simulink model "TosAeb"  (R2021a)
settings: Solver=FixedStepDiscrete  StartTime=0.0  StopTime=inf  FixedStep=0.01  RelTol=1e-3
undefined symbols (not defined in the file or in its folder): ACCModSts, AccSts1, AEB_ObjectMovingOrientation, AEB_ObjectMovingState, CN_BTCActive, CN_CurrentGearR, CN_CurrentGearR_DHT, CN_PTCActive, CN_VDCActive, CN_VSC_EspOff, CN_VSC_FCW_AEB_Error, CN_VSC_FCW_AEB_NoDispay, CN_VSC_FCW_AEB_PerfmDgdtn, CN_VSC_FCW_AEB_TempUnAvlbl, CN_VSC_VehStandstill, CNaccelAvoidMax, CNaccelAvoidMin, CNbrakePedalPress, CNcomfortStrLeftAccelMax, CNcomfortStrLeftAccelMin, CNcomfortStrRightAccelMax, CNcomfortStrRightAccelMin, CNDrvDoorOpen, CNDrvModeEco, CNDrvModeNorm, CNDrvModeOther, CNDrvModeSport, CNDrvSBRreminder, CNNull, CNobjInvalidID, CNPassDoorOpen, CNPassSBRreminder, CNPEBSAccelNegInf, CNPEBSAccelNull, CNPEBSTimeInf, CNPEBSTimeNull, CNSTOPMAX, CNSTOPNULL, CNsubjWidth, CNSwtOFF, CNSwtON, CNTTCMAX, CNTTCNULL, CNVehConfigAEBCycNone, CNVehConfigAEBNone, CNVehConfigAEBPedNone, CNVehConfigFCWNone, CNVehConfigJANone, CollisionSeverity, FALSE, FCWSnvty, JACycObjCalcInfoDefaultParams, JAPedObjCalcInfoDefaultParams, JAVehObjCalcInfoDefaultParams, latCycObjCalcInfoDefaultParams, latPedObjCalcInfoDefaultParams, longObjCalcInfoDefaultParams, ModSts, movingStatus, ObjectType ... (+1112)
libraries: simulink x3031, AEBSFunctionLib x50, Ta_Library x33, AEBSStateMachineLib x23, Mylibrary x12, simulink_extras x7, ObjSelect_lib x3, dspstat3 x2
(part 42 of 102)

== system TosAeb/FcwAebJa_Step_sys/ALG_AEBSystem/ALG_AEBController/AEB_FuncCondCalcApp/LongFunctionCondCalc/vehLatentWarningSuppAborCondFulfilled/vehLatentWarningAborCondCalc/vehLatentWarningSituationRelAborCondCalc ==
  subjVel : Inport
  dxFrontBumper : Inport  Port=2
  Compare To Constant : simulink/Logic and Bit Operations/Compare To Constant  relop===  const=0  OutDataTypeStr=boolean
  Compare To Constant1 : simulink/Logic and Bit Operations/Compare To Constant  relop=>  const=P_AEB_Func_vehLatentWarningTimeGapCounterThres  OutDataTypeStr=boolean
  Compare To Constant2 : simulink/Logic and Bit Operations/Compare To Constant  relop=>  const=P_AEB_Func_vehLatentWarningTimeGapDurationAborThres  OutDataTypeStr=boolean
  If : If  IfExpression=u1
  If Action Subsystem : Subsystem  TreatAsAtomicUnit=on
  If Action Subsystem1 : Subsystem  TreatAsAtomicUnit=on
  Logical Operator2 : Logic  AllPortsSameDT=off  OutDataTypeStr=boolean
  Merge : Merge
  timer : Subsystem
  vehLatentWarningSituationRelAborCondFulfilled : Outport
  wires:
  Logical Operator2/1 -> vehLatentWarningSituationRelAborCondFulfilled/1
  Compare To Constant1/1 -> timer/1(cond), Logical Operator2/1
  If/2 -> If Action Subsystem1/Action
  If Action Subsystem/1(latentWarningTimeGap) -> Merge/1
  If Action Subsystem1/1(latentWarningTimeGap) -> Merge/2
  dxFrontBumper/1 -> If Action Subsystem1/1(dxFrontBumper)
  subjVel/1 -> If Action Subsystem1/2(subjVel), Compare To Constant/1
  Compare To Constant/1 -> If/1
  If/1 -> If Action Subsystem/Action
  Merge/1 -> Compare To Constant1/1
  timer/1(time) -> Compare To Constant2/1
  Compare To Constant2/1 -> Logical Operator2/2

== system TosAeb/FcwAebJa_Step_sys/ALG_AEBSystem/ALG_AEBController/AEB_FuncCondCalcApp/LongFunctionCondCalc/vehLatentWarningSuppAborCondFulfilled/vehLatentWarningAborCondCalc/vehLatentWarningVehRelActiveCondCalc ==
  vehStateCalcLatentWarningAborFlg : Inport
  subjVel : Inport  Port=2
  ACCModSts : Inport  Port=3
  ACCStateJudge : Subsystem
  vehLatentWarningVehRelAborCondJudge : Subsystem
  vehLatentWarningVehRelAborCondFulfilled : Outport
  wires:
  ACCModSts/1 -> ACCStateJudge/1(ACCModSts)
  subjVel/1 -> vehLatentWarningVehRelAborCondJudge/2(subjVel)
  vehLatentWarningVehRelAborCondJudge/1(vehLatentWarningVehRelAborCondFulfilled) -> vehLatentWarningVehRelAborCondFulfilled/1
  vehStateCalcLatentWarningAborFlg/1 -> vehLatentWarningVehRelAborCondJudge/1(vehStateCalcLatentWarningAborFlg)
  ACCStateJudge/1(ACCStateSuppCondFlg) -> vehLatentWarningVehRelAborCondJudge/3(ACCStateSuppCondFlg)

== system TosAeb/FcwAebJa_Step_sys/ALG_AEBSystem/ALG_AEBController/AEB_FuncCondCalcApp/LongFunctionCondCalc/vehLatentWarningSuppAborCondFulfilled/vehLatentWarningSuppCondCalc/vehLatentWarningObjRelActiveCondCalc ==
  ObjectMovingState : Inport
  ObjectType : Inport  Port=2
  ObjectMovingOrientation : Inport  Port=3
  ObjectID : Inport  Port=4
  ObjectExistProb : Inport  Port=5
  ObjectIsObstacleProb : Inport  Port=6
  Compare To Constant : simulink/Logic and Bit Operations/Compare To Constant  relop===  const=AEB_ObjectMovingState.Still  OutDataTypeStr=boolean
  Compare To Constant1 : simulink/Logic and Bit Operations/Compare To Constant  relop===  const=AEB_ObjectMovingState.Moving  OutDataTypeStr=boolean
  Compare To Constant12 : simulink/Logic and Bit Operations/Compare To Constant  relop===  const=FALSE  OutDataTypeStr=boolean
  Compare To Constant14 : simulink/Logic and Bit Operations/Compare To Constant  relop===  const=ObjectTypeCopy.PEDESTRIAN  OutDataTypeStr=boolean
  Compare To Constant15 : simulink/Logic and Bit Operations/Compare To Constant  relop===  const=ObjectTypeCopy.BICYCLE  OutDataTypeStr=boolean
  Compare To Constant2 : simulink/Logic and Bit Operations/Compare To Constant  relop===  const=AEB_ObjectMovingState.Stopped  OutDataTypeStr=boolean
  Compare To Constant3 : simulink/Logic and Bit Operations/Compare To Constant  relop===  const=AEB_ObjectMovingOrientation.SameDir  OutDataTypeStr=boolean
  Compare To Constant4 : simulink/Logic and Bit Operations/Compare To Constant  relop=~=  const=CNobjInvalidID  OutDataTypeStr=boolean
  Compare To Constant5 : simulink/Logic and Bit Operations/Compare To Constant  relop=>  const=P_AEB_Func_vehLatentWarningObjObstacleProbActivationThres  OutDataTypeStr=boolean
  Compare To Constant6 : simulink/Logic and Bit Operations/Compare To Constant  relop=~=  const=CNobjInvalidID  OutDataTypeStr=boolean
  Logical Operator : Logic  Operator=OR  Inputs=3  AllPortsSameDT=off  OutDataTypeStr=boolean
  Logical Operator1 : Logic  AllPortsSameDT=off  OutDataTypeStr=boolean
  Logical Operator2 : Logic  AllPortsSameDT=off  OutDataTypeStr=boolean
  Logical Operator5 : Logic  Inputs=5  AllPortsSameDT=off  OutDataTypeStr=boolean
  Logical Operator6 : Logic  Operator=OR  AllPortsSameDT=off  OutDataTypeStr=boolean
  Product : Product
  Relational Operator : RelationalOperator  Operator===  OutDataTypeStr=boolean  RndMeth=Simplest
  Unit Delay : UnitDelay  SampleTime=-1  HasFrameUpgradeWarning=on
  vehLatentWarningObjRelActiveCondFulfilled : Outport
  wires:
  Compare To Constant15/1 -> Logical Operator6/2
  Compare To Constant14/1 -> Logical Operator6/1
  ObjectMovingState/1 -> Compare To Constant/1, Compare To Constant2/1, Compare To Constant1/1
  Compare To Constant2/1 -> Logical Operator/2
  Compare To Constant1/1 -> Logical Operator/1
  ObjectMovingOrientation/1 -> Compare To Constant3/1
  ObjectID/1 -> Compare To Constant6/1, Unit Delay/1, Relational Operator/1, Compare To Constant4/1
  Unit Delay/1 -> Relational Operator/2
  ObjectExistProb/1 -> Product/1
  ObjectIsObstacleProb/1 -> Product/2
  Compare To Constant6/1 -> Logical Operator2/1
  Compare To Constant5/1 -> Logical Operator2/2
  Product/1 -> Compare To Constant5/1
  Compare To Constant/1 -> Logical Operator/3
  Relational Operator/1 -> Logical Operator1/2
  Compare To Constant4/1 -> Logical Operator1/1
  Logical Operator5/1 -> vehLatentWarningObjRelActiveCondFulfilled/1  "vehPreWarningObjRelActiveCondFulfilled"
  Compare To Constant3/1 -> Logical Operator5/3  "objMovingOrientationCondFulfilled"
  Logical Operator2/1 -> Logical Operator5/5  "objQualityCondFulfilled"
  Logical Operator6/1 -> Compare To Constant12/1  "pedCycObjCondSuppFlg"
  Logical Operator/1 -> Logical Operator5/1  "objMovingStateCondFulfilled"
  Logical Operator1/1 -> Logical Operator5/4  "objIDCondFulfilled"
  ObjectType/1 -> Compare To Constant14/1, Compare To Constant15/1
  Compare To Constant12/1 -> Logical Operator5/2  "pedCycReleventCondFulfilled"

== system TosAeb/FcwAebJa_Step_sys/ALG_AEBSystem/ALG_AEBController/AEB_FuncCondCalcApp/LongFunctionCondCalc/vehLatentWarningSuppAborCondFulfilled/vehLatentWarningSuppCondCalc/vehLatentWarningSituationRelActiveCondCalc ==
  subjVel : Inport
  dxFrontBumper : Inport  Port=2
  FCWSnvty : Inport  Port=3
  Compare To Constant : simulink/Logic and Bit Operations/Compare To Constant  relop=<  const=0.1  OutDataTypeStr=boolean
  Constant : Constant  Value=Ts
  Constant1 : Constant  Value=30
  If : If  IfExpression=u1
  If Action Subsystem : Subsystem  TreatAsAtomicUnit=on
  If Action Subsystem1 : Subsystem  TreatAsAtomicUnit=on
  Logical Operator2 : Logic  AllPortsSameDT=off  OutDataTypeStr=boolean
  Merge : Merge
  Relational Operator : RelationalOperator  Operator=<  OutDataTypeStr=boolean  RndMeth=Simplest
  Relational Operator1 : RelationalOperator  Operator=>  OutDataTypeStr=boolean  RndMeth=Simplest
  TimeThesCalc : Subsystem
  timer1 : AEBSFunctionLib/timer  SystemSampleTime=-1  VariantActivationTime=update diagram  Latency=0  AutoFrameSizeCalculation=off
  vehLatentWarningSituationRelActiveCondFulfilled : Outport
  wires:
  Constant1/1 -> timer1/3
  Constant/1 -> timer1/2
  TimeThesCalc/2(prolongTime) -> Relational Operator1/2
  timer1/1 -> Relational Operator1/1
  TimeThesCalc/1(timeGapThes) -> Relational Operator/2
  Merge/1 -> Relational Operator/1
  FCWSnvty/1 -> TimeThesCalc/1(FCWSnvty)
  Relational Operator1/1 -> Logical Operator2/2
  Relational Operator/1 -> Logical Operator2/1, timer1/1
  If Action Subsystem1/1(latentWarningTimeGap) -> Merge/2
  If Action Subsystem/1(latentWarningTimeGap) -> Merge/1
  subjVel/1 -> If Action Subsystem1/2(subjVel), Compare To Constant/1
  dxFrontBumper/1 -> If Action Subsystem1/1(dxFrontBumper)
  If/2 -> If Action Subsystem1/Action
  If/1 -> If Action Subsystem/Action
  Compare To Constant/1 -> If/1
  Logical Operator2/1 -> vehLatentWarningSituationRelActiveCondFulfilled/1  "vehLatentWarningSituationRelActiveCondFulfilled"

== system TosAeb/FcwAebJa_Step_sys/ALG_AEBSystem/ALG_AEBController/AEB_FuncCondCalcApp/LongFunctionCondCalc/vehLatentWarningSuppAborCondFulfilled/vehLatentWarningSuppCondCalc/vehLatentWarningSuppCondFinalJudge ==
  vehLatentWarningObjRelActiveCondFulfilled : Inport
  vehLatentWarningVehRelActiveCondFulfilled : Inport  Port=2
  vehLatentWarningSituationRelActiveCondFulfilled : Inport  Port=3
  Compare To Constant : simulink/Logic and Bit Operations/Compare To Constant  relop===  const=FALSE  OutDataTypeStr=boolean
  Compare To Constant1 : simulink/Logic and Bit Operations/Compare To Constant  relop===  const=FALSE  OutDataTypeStr=boolean
  Compare To Constant2 : simulink/Logic and Bit Operations/Compare To Constant  relop===  const=FALSE  OutDataTypeStr=boolean
  Logical Operator : Logic  Operator=OR  Inputs=3  AllPortsSameDT=off  OutDataTypeStr=boolean
  vehLatentWarningAborCondFulfilled : Outport
  wires:
  Compare To Constant2/1 -> Logical Operator/3
  Compare To Constant1/1 -> Logical Operator/2
  Compare To Constant/1 -> Logical Operator/1
  vehLatentWarningVehRelActiveCondFulfilled/1 -> Compare To Constant1/1
  vehLatentWarningObjRelActiveCondFulfilled/1 -> Compare To Constant/1
  vehLatentWarningSituationRelActiveCondFulfilled/1 -> Compare To Constant2/1
  Logical Operator/1 -> vehLatentWarningAborCondFulfilled/1

== system TosAeb/FcwAebJa_Step_sys/ALG_AEBSystem/ALG_AEBController/AEB_FuncCondCalcApp/LongFunctionCondCalc/vehLatentWarningSuppAborCondFulfilled/vehLatentWarningSuppCondCalc/vehLatentWarningVehRelActiveCondCalc ==
  subjVel : Inport
  ACCModSts : Inport  Port=2
  vehStateCalcLatentWarningSuppFlg : Inport  Port=3
  ACCStateJudge : Subsystem
  subjVelWork : Subsystem
  vehLatentWarningVehRelActiveCondJudge : Subsystem
  vehLatentWarningVehRelActiveCondFulfilled : Outport
  wires:
  ACCModSts/1 -> ACCStateJudge/1(ACCModSts)
  subjVelWork/1(subjVelWorkMax) -> vehLatentWarningVehRelActiveCondJudge/2(subjVelWorkMax)
  subjVelWork/2(subjVelWorkMin) -> vehLatentWarningVehRelActiveCondJudge/3(subjVelWorkMin)
  ACCStateJudge/1(ACCStateSuppCondFlg) -> vehLatentWarningVehRelActiveCondJudge/4(ACCStateSuppCondFlg)
  subjVel/1 -> vehLatentWarningVehRelActiveCondJudge/1(subjVel)
  vehStateCalcLatentWarningSuppFlg/1 -> vehLatentWarningVehRelActiveCondJudge/5(vehStateCalcLatentWarningSuppFlg)
  vehLatentWarningVehRelActiveCondJudge/1(vehLatentWarningVehRelActiveCondFulfilled) -> vehLatentWarningVehRelActiveCondFulfilled/1

== system TosAeb/FcwAebJa_Step_sys/ALG_AEBSystem/ALG_AEBController/AEB_FuncCondCalcApp/LongFunctionCondCalc/vehPreWarningSuppAborCondFulfilled/vehPreWarningAborCondCalc/vehPreWarningAborCondFinalJudge ==
  vehPreWarningObjRelAborCondFulfilled : Inport
  vehPreWarningVehRelAborCondFulfilled : Inport  Port=2
  vehPreWarningSituationRelAborCondFulfilled : Inport  Port=3
  Compare To Constant : simulink/Logic and Bit Operations/Compare To Constant  relop===  const=TRUE  OutDataTypeStr=boolean
  Compare To Constant1 : simulink/Logic and Bit Operations/Compare To Constant  relop===  const=TRUE  OutDataTypeStr=boolean
  Compare To Constant2 : simulink/Logic and Bit Operations/Compare To Constant  relop===  const=TRUE  OutDataTypeStr=boolean
  Logical Operator : Logic  Operator=OR  Inputs=3  AllPortsSameDT=off  OutDataTypeStr=boolean
  vehPreWarningAborCond : Outport
  wires:
  Compare To Constant2/1 -> Logical Operator/3
  Compare To Constant1/1 -> Logical Operator/2
  Compare To Constant/1 -> Logical Operator/1
  Logical Operator/1 -> vehPreWarningAborCond/1
  vehPreWarningSituationRelAborCondFulfilled/1 -> Compare To Constant2/1
  vehPreWarningObjRelAborCondFulfilled/1 -> Compare To Constant/1
  vehPreWarningVehRelAborCondFulfilled/1 -> Compare To Constant1/1

== system TosAeb/FcwAebJa_Step_sys/ALG_AEBSystem/ALG_AEBController/AEB_FuncCondCalcApp/LongFunctionCondCalc/vehPreWarningSuppAborCondFulfilled/vehPreWarningAborCondCalc/vehPreWarningObjRelAborCondCalc ==
  ObjectID : Inport
  Compare To Constant4 : simulink/Logic and Bit Operations/Compare To Constant  relop===  const=CNobjInvalidID  OutDataTypeStr=boolean
  Logical Operator1 : Logic  Operator=OR  AllPortsSameDT=off  OutDataTypeStr=boolean
  Relational Operator : RelationalOperator  Operator=~=  OutDataTypeStr=boolean  RndMeth=Simplest
  Unit Delay : UnitDelay  SampleTime=-1  HasFrameUpgradeWarning=on
  vehPreWarningObjRelAborCondFulfilled : Outport
  wires:
  ObjectID/1 -> Unit Delay/1, Relational Operator/1, Compare To Constant4/1
  Unit Delay/1 -> Relational Operator/2
  Relational Operator/1 -> Logical Operator1/2
  Compare To Constant4/1 -> Logical Operator1/1
  Logical Operator1/1 -> vehPreWarningObjRelAborCondFulfilled/1  "vehPreWarningObjRelAborCondFulfilled"

== system TosAeb/FcwAebJa_Step_sys/ALG_AEBSystem/ALG_AEBController/AEB_FuncCondCalcApp/LongFunctionCondCalc/vehPreWarningSuppAborCondFulfilled/vehPreWarningAborCondCalc/vehPreWarningSituationRelAborCondCalc ==
  longCollisionSeverity : Inport
  Compare To Constant : simulink/Logic and Bit Operations/Compare To Constant  relop=~=  const=CollisionSeverity.Low  OutDataTypeStr=boolean
  Compare To Constant1 : simulink/Logic and Bit Operations/Compare To Constant  relop=~=  const=CollisionSeverity.Medium  OutDataTypeStr=boolean
  Compare To Constant2 : simulink/Logic and Bit Operations/Compare To Constant  relop=~=  const=CollisionSeverity.High  OutDataTypeStr=boolean
  Logical Operator : Logic  Inputs=3  AllPortsSameDT=off  OutDataTypeStr=boolean
  vehPreWarningSituationRelAborCondFulfilled : Outport
  wires:
  longCollisionSeverity/1 -> Compare To Constant/1, Compare To Constant2/1, Compare To Constant1/1
  Compare To Constant/1 -> Logical Operator/1
  Compare To Constant1/1 -> Logical Operator/2
  Compare To Constant2/1 -> Logical Operator/3
  Logical Operator/1 -> vehPreWarningSituationRelAborCondFulfilled/1  "vehPreWarningSituationRelAborCondFulfilled"

== system TosAeb/FcwAebJa_Step_sys/ALG_AEBSystem/ALG_AEBController/AEB_FuncCondCalcApp/LongFunctionCondCalc/vehPreWarningSuppAborCondFulfilled/vehPreWarningAborCondCalc/vehPreWarningVehRelActiveCondCalc ==
  SteerWheelAngle : Inport
  SteerWheelSpd : Inport  Port=2
  ESPmasterCylindBrakePress : Inport  Port=3
  vehStateCalcPreWarningAborFlg : Inport  Port=4
  EBAFuncImpArb : Inport  Port=5
  DriverCondJudge : Subsystem
  vehPreWarningVehRelAborCondJudge : Subsystem
  vehPreWarningVehRelAborCondFulfilled : Outport
  wires:
  EBAFuncImpArb/1 -> vehPreWarningVehRelAborCondJudge/3(EBAFuncImpArb)
  SteerWheelAngle/1 -> DriverCondJudge/1(SteerWheelAngle)
  SteerWheelSpd/1 -> DriverCondJudge/2(SteerWheelSpd)
  ESPmasterCylindBrakePress/1 -> DriverCondJudge/3(AccrPedlPosnDiagc)
  DriverCondJudge/1(driverAborCondFlg) -> vehPreWarningVehRelAborCondJudge/1(driverAborCondFlg)
  vehStateCalcPreWarningAborFlg/1 -> vehPreWarningVehRelAborCondJudge/2(vehStateCalcPreWarningAborFlg)
  vehPreWarningVehRelAborCondJudge/1(vehPreWarningVehRelAborCondFulfilled) -> vehPreWarningVehRelAborCondFulfilled/1  "vehPreWarningVehRelAborCondFulfilled"

== system TosAeb/FcwAebJa_Step_sys/ALG_AEBSystem/ALG_AEBController/AEB_FuncCondCalcApp/LongFunctionCondCalc/vehPreWarningSuppAborCondFulfilled/vehPreWarningSuppCondCalc/vehPreWarningObjRelActiveCondCalc ==
  ObjectMovingState : Inport
  dz : Inport  Port=2
  ObjectTypeCameraConfirmed : Inport  Port=3
  AEB_PedResp : Inport  Port=4
  ObjectType : Inport  Port=5  OutDataTypeStr=Enum: ObjectTypeCopy
  subjCurvTraj : Inport  Port=6
  subjLatAccel : Inport  Port=7
  subjVel : Inport  Port=8
  vehConfigAEBCyc : Inport  Port=9
  vehConfigAEBPed : Inport  Port=10
  ObjectMovingOrientation : Inport  Port=11
  ObjectID : Inport  Port=12
  ObjectExistProb : Inport  Port=13
  ObjectIsObstacleProb : Inport  Port=14
  dxVariance : Inport  Port=15
  dxFrontBumper : Inport  Port=16
  ObjFusQuality : Inport  Port=17
  Compare To Constant : simulink/Logic and Bit Operations/Compare To Constant  relop===  const=AEB_ObjectMovingState.Still  OutDataTypeStr=boolean
  Compare To Constant1 : simulink/Logic and Bit Operations/Compare To Constant  relop===  const=AEB_ObjectMovingState.Moving  OutDataTypeStr=boolean
  Compare To Constant10 : simulink/Logic and Bit Operations/Compare To Constant  relop=<  const=P_AEB_Func_vehPreWarningDxActivationThres  OutDataTypeStr=boolean
  Compare To Constant11 : simulink/Logic and Bit Operations/Compare To Constant  relop===  const=TRUE  OutDataTypeStr=boolean
  Compare To Constant12 : simulink/Logic and Bit Operations/Compare To Constant  relop===  const=FALSE  OutDataTypeStr=boolean
  Compare To Constant14 : simulink/Logic and Bit Operations/Compare To Constant  relop===  const=AEB_ObjectMovingState.Still  OutDataTypeStr=boolean
  Compare To Constant2 : simulink/Logic and Bit Operations/Compare To Constant  relop===  const=AEB_ObjectMovingState.Stopped  OutDataTypeStr=boolean
  Compare To Constant3 : simulink/Logic and Bit Operations/Compare To Constant  relop===  const=AEB_ObjectMovingOrientation.SameDir  OutDataTypeStr=boolean
  Compare To Constant4 : simulink/Logic and Bit Operations/Compare To Constant  relop=~=  const=CNobjInvalidID  OutDataTypeStr=boolean
  Compare To Constant5 : simulink/Logic and Bit Operations/Compare To Constant  relop=>  const=P_AEB_Func_vehPreWarningObjObstacleProbActivationThres  OutDataTypeStr=boolean
  Compare To Constant6 : simulink/Logic and Bit Operations/Compare To Constant  relop=~=  const=CNobjInvalidID  OutDataTypeStr=boolean
  Compare To Constant7 : simulink/Logic and Bit Operations/Compare To Constant  relop===  const=TRUE  OutDataTypeStr=boolean
  Compare To Constant8 : simulink/Logic and Bit Operations/Compare To Constant  relop=<  const=P_AEB_Func_vehPreWarningObjHeightActivationThres  OutDataTypeStr=boolean
  Compare To Constant9 : simulink/Logic and Bit Operations/Compare To Constant  relop=<  const=P_AEB_Func_vehPreWarningDxVarActivationThres  OutDataTypeStr=boolean
  Constant : Constant  Value=TRUE
  Constant1 : Constant  Value=P_AEB_Func_vehPreWarningSubjLatAccelActivationThresForPedCyc
  Constant2 : Constant  Value=P_AEB_Func_vehPreWarningSubjCurvTrajActivationThresForPedCyc
  Constant3 : Constant  Value=P_AEB_Func_vehPreWarningSubjVelActivationThresForPedCyc
  Logical Operator : Logic  Operator=OR  Inputs=3  AllPortsSameDT=off  OutDataTypeStr=boolean
  Logical Operator1 : Logic  AllPortsSameDT=off  OutDataTypeStr=boolean
  Logical Operator2 : Logic  AllPortsSameDT=off  OutDataTypeStr=boolean
  Logical Operator5 : Logic  Inputs=10  AllPortsSameDT=off  OutDataTypeStr=boolean
  PedCycObjCondSuppJudgeFlow : AEBSFunctionLib/PedCycObjCondSuppJudgeFlow  SystemSampleTime=-1  VariantActivationTime=update diagram  Latency=0  AutoFrameSizeCalculation=off
  Product : Product
  Relational Operator : RelationalOperator  Operator===  OutDataTypeStr=boolean  RndMeth=Simplest
  Switch : Switch  Criteria=u2 ~= 0
  Unit Delay : UnitDelay  SampleTime=-1  HasFrameUpgradeWarning=on
  vehPreWarningObjRelActiveCondFulfilled : Outport
  wires:
  Compare To Constant11/1 -> Logical Operator5/10  "ObjFusQuality"
  ObjFusQuality/1 -> Compare To Constant11/1
  subjLatAccel/1 -> PedCycObjCondSuppJudgeFlow/4
  subjVel/1 -> PedCycObjCondSuppJudgeFlow/5
  vehConfigAEBCyc/1 -> PedCycObjCondSuppJudgeFlow/6
  vehConfigAEBPed/1 -> PedCycObjCondSuppJudgeFlow/7
  AEB_PedResp/1 -> PedCycObjCondSuppJudgeFlow/8
  Constant3/1 -> PedCycObjCondSuppJudgeFlow/9
  Constant2/1 -> PedCycObjCondSuppJudgeFlow/10
  Constant1/1 -> PedCycObjCondSuppJudgeFlow/11
  subjCurvTraj/1 -> PedCycObjCondSuppJudgeFlow/3
  ObjectType/1 -> PedCycObjCondSuppJudgeFlow/2
  Constant/1 -> Switch/3
  Compare To Constant14/1 -> Switch/2
  Compare To Constant8/1 -> Switch/1
  Compare To Constant7/1 -> Logical Operator5/3  "FusionCondFulfilled"
  Compare To Constant12/1 -> Logical Operator5/4  "pedCycReleventCondFulfilled"
  ObjectTypeCameraConfirmed/1 -> Compare To Constant7/1, PedCycObjCondSuppJudgeFlow/1
  Compare To Constant9/1 -> Logical Operator5/8  "dxVarCondFulfilled"
  Logical Operator1/1 -> Logical Operator5/6  "objIDCondFulfilled"
  Logical Operator/1 -> Logical Operator5/1  "objMovingStateCondFulfilled"
  Compare To Constant10/1 -> Logical Operator5/9  "dxRelCondFulfilled"
  PedCycObjCondSuppJudgeFlow/1 -> Compare To Constant12/1  "pedCycObjCondSuppFlg"
  Logical Operator2/1 -> Logical Operator5/7  "objQualityCondFulfilled"
  Compare To Constant3/1 -> Logical Operator5/5  "objMovingOrientationCondFulfilled"
  Switch/1 -> Logical Operator5/2  "objHeightInfoFulfilled"
  Logical Operator5/1 -> vehPreWarningObjRelActiveCondFulfilled/1  "vehPreWarningObjRelActiveCondFulfilled"
  Compare To Constant4/1 -> Logical Operator1/1
  Relational Operator/1 -> Logical Operator1/2
  Compare To Constant/1 -> Logical Operator/3
  dz/1 -> Compare To Constant8/1
  dxVariance/1 -> Compare To Constant9/1
  dxFrontBumper/1 -> Compare To Constant10/1
  Product/1 -> Compare To Constant5/1
  Compare To Constant5/1 -> Logical Operator2/2
  Compare To Constant6/1 -> Logical Operator2/1
  ObjectIsObstacleProb/1 -> Product/2
  ObjectExistProb/1 -> Product/1
  Unit Delay/1 -> Relational Operator/2
  ObjectID/1 -> Compare To Constant4/1, Relational Operator/1, Unit Delay/1, Compare To Constant6/1
  ObjectMovingOrientation/1 -> Compare To Constant3/1
  Compare To Constant1/1 -> Logical Operator/1
  Compare To Constant2/1 -> Logical Operator/2
  ObjectMovingState/1 -> Compare To Constant14/1, Compare To Constant/1, Compare To Constant2/1, Compare To Constant1/1

== system TosAeb/FcwAebJa_Step_sys/ALG_AEBSystem/ALG_AEBController/AEB_FuncCondCalcApp/LongFunctionCondCalc/vehPreWarningSuppAborCondFulfilled/vehPreWarningSuppCondCalc/vehPreWarningSituationRelActiveCondCalc ==
  longCollisionSeverity : Inport  OutDataTypeStr=Enum: CollisionSeverity
  overlapRatio : Inport  Port=2
  dxFrontBumper : Inport  Port=3
  probEscape : Inport  Port=4
  subjCurvTraj : Inport  Port=5
  TTC : Inport  Port=6
  FcwHMISetting : Inport  Port=7
  subjVel : Inport  Port=8  OutDataTypeStr=single
  ACCModSts : Inport  Port=9
  AEB_Func_VehPreWarning_TTC_Thres1 : Lookup_n-D  Commented=on  NumberOfTableDimensions=1  BreakpointsForDimension1=P_AEB_Func_vehPreWarning_TTC_ThresX  Table=P_AEB_Func_vehPreWarning_TTC_ThresY  ExtrapMethod=Clip  InternalRulePriority=Speed  RndMeth=Simplest  InputSameDT=on
  IsTtcCondFulfilledCalc : Subsystem
  Subsystem1 : Subsystem
  vehPreWarningSituationRelActiveCondFulfilled : Outport
  wires:
  TTC/1 -> IsTtcCondFulfilledCalc/1(TTC)
  subjVel/1 -> IsTtcCondFulfilledCalc/3(subjVel)
  FcwHMISetting/1 -> IsTtcCondFulfilledCalc/2(FcwHMISetting)
  longCollisionSeverity/1 -> Subsystem1/1(longCollisionSeverity)
  overlapRatio/1 -> Subsystem1/2(overlapRatio)
  dxFrontBumper/1 -> Subsystem1/3(dxFrontBumper)
  probEscape/1 -> Subsystem1/4(probEscape)
  subjCurvTraj/1 -> Subsystem1/5(subjCurvTraj)
  IsTtcCondFulfilledCalc/1(IsTtcCondFulfilled) -> Subsystem1/6(IsTtcCondFulfilled)  "IsTtcCondFulfilled"
  Subsystem1/1(vehPreWarningSituationRelActiveCondFulfilled) -> vehPreWarningSituationRelActiveCondFulfilled/1
  ACCModSts/1 -> IsTtcCondFulfilledCalc/4(ACCModSts)

== system TosAeb/FcwAebJa_Step_sys/ALG_AEBSystem/ALG_AEBController/AEB_FuncCondCalcApp/LongFunctionCondCalc/vehPreWarningSuppAborCondFulfilled/vehPreWarningSuppCondCalc/vehPreWarningSuppCondFinalJudge ==
  vehPreWarningObjRelActiveCondFulfilled : Inport
  vehPreWarningVehRelActiveCondFulfilled : Inport  Port=2
  vehPreWarningSituationRelActiveCondFulfilled : Inport  Port=3
  Compare To Constant1 : simulink/Logic and Bit Operations/Compare To Constant  relop===  const=FALSE  OutDataTypeStr=boolean
  Compare To Constant2 : simulink/Logic and Bit Operations/Compare To Constant  relop===  const=FALSE  OutDataTypeStr=boolean
  Compare To Constant5 : simulink/Logic and Bit Operations/Compare To Constant  relop===  const=FALSE  OutDataTypeStr=boolean
  Logical Operator : Logic  Operator=OR  Inputs=3  AllPortsSameDT=off  OutDataTypeStr=boolean
  vehPreWarningSuppCond : Outport
  wires:
  Compare To Constant2/1 -> Logical Operator/3
  Compare To Constant1/1 -> Logical Operator/2
  Compare To Constant5/1 -> Logical Operator/1
  Logical Operator/1 -> vehPreWarningSuppCond/1
  vehPreWarningSituationRelActiveCondFulfilled/1 -> Compare To Constant2/1
  vehPreWarningObjRelActiveCondFulfilled/1 -> Compare To Constant5/1
  vehPreWarningVehRelActiveCondFulfilled/1 -> Compare To Constant1/1

== system TosAeb/FcwAebJa_Step_sys/ALG_AEBSystem/ALG_AEBController/AEB_FuncCondCalcApp/LongFunctionCondCalc/vehPreWarningSuppAborCondFulfilled/vehPreWarningSuppCondCalc/vehPreWarningVehRelActiveCondCalc ==
  subjVel : Inport
  ObjectMovingState : Inport  Port=2
  vehStateCalcPreWarningSuppFlg : Inport  Port=3
  SteerWheelAngle : Inport  Port=4
  SteerWheelSpd : Inport  Port=5
  VehConfigIbooster : Inport  Port=6
  ESPmasterCylindBrakePress : Inport  Port=7
  BrakePedalStatus_G : Inport  Port=8
  IB_BrkPedalAppld : Inport  Port=9
  AccrPedlPosnDiagc : Inport  Port=10
  DriverCondJudge : Subsystem
  subjVelWork : Subsystem
  vehPreWarningVehRelActiveCondJudge : Subsystem
  vehPreWarningVehRelActiveCondFulfilled : Outport
  wires:
  VehConfigIbooster/1 -> DriverCondJudge/3(VehConfigIbooster)
  IB_BrkPedalAppld/1 -> DriverCondJudge/6(IB_BrkPedalAppld)
  AccrPedlPosnDiagc/1 -> DriverCondJudge/7(AccrPedlPosnDiagc)
  BrakePedalStatus_G/1 -> DriverCondJudge/5(BrakePedalStatus_G)
  ESPmasterCylindBrakePress/1 -> DriverCondJudge/4(ESPmasterCylindBrakePress)
  SteerWheelSpd/1 -> DriverCondJudge/2(SteerWheelSpd)
  SteerWheelAngle/1 -> DriverCondJudge/1(SteerWheelAngle)
  vehPreWarningVehRelActiveCondJudge/1(vehPreWarningVehRelActiveCondFulfilled) -> vehPreWarningVehRelActiveCondFulfilled/1  "vehPreWarningVehRelActiveCondFulfilled"
  vehStateCalcPreWarningSuppFlg/1 -> vehPreWarningVehRelActiveCondJudge/5(vehStateCalcPreWarningSuppFlg)
  subjVel/1 -> vehPreWarningVehRelActiveCondJudge/1(subjVel)
  DriverCondJudge/1(driverSuppCondFlg) -> vehPreWarningVehRelActiveCondJudge/4(driverSuppCondFlg)
  subjVelWork/2(subjVelWorkMin) -> vehPreWarningVehRelActiveCondJudge/3(subjVelWorkMin)
  subjVelWork/1(subjVelWorkMax) -> vehPreWarningVehRelActiveCondJudge/2(subjVelWorkMax)
  ObjectMovingState/1 -> subjVelWork/1(ObjectMovingState)

== system TosAeb/FcwAebJa_Step_sys/ALG_AEBSystem/ALG_AEBController/AEB_FuncCondCalcApp/LongFunctionCondCalc/vehPrefillSuppAborCondCalc/vehPrefillAborCondCalc/vehPrefillAborCondFinalJudge ==
  vehPrefillObjRelAborCondFulfilled : Inport
  vehPrefillVehRelAborCondFulfilled : Inport  Port=2
  vehPrefillSituationRelAborCondFulfilled : Inport  Port=3
  Compare To Constant1 : simulink/Logic and Bit Operations/Compare To Constant  relop===  const=TRUE  OutDataTypeStr=boolean
  Compare To Constant2 : simulink/Logic and Bit Operations/Compare To Constant  relop===  const=TRUE  OutDataTypeStr=boolean
  Compare To Constant3 : simulink/Logic and Bit Operations/Compare To Constant  relop===  const=TRUE  OutDataTypeStr=boolean
  Logical Operator : Logic  Operator=OR  Inputs=3  AllPortsSameDT=off  OutDataTypeStr=boolean
  vehPrefillAborCondFulfilled : Outport
  wires:
  Compare To Constant3/1 -> Logical Operator/3
  Compare To Constant2/1 -> Logical Operator/2
  Compare To Constant1/1 -> Logical Operator/1
  Logical Operator/1 -> vehPrefillAborCondFulfilled/1  "vehPrefillAborCondFulfilled"
  vehPrefillSituationRelAborCondFulfilled/1 -> Compare To Constant3/1
  vehPrefillObjRelAborCondFulfilled/1 -> Compare To Constant1/1
  vehPrefillVehRelAborCondFulfilled/1 -> Compare To Constant2/1

== system TosAeb/FcwAebJa_Step_sys/ALG_AEBSystem/ALG_AEBController/AEB_FuncCondCalcApp/LongFunctionCondCalc/vehPrefillSuppAborCondCalc/vehPrefillAborCondCalc/vehPrefillObjRelAborCondCalc ==
  ObjectID : Inport
  Compare To Constant4 : simulink/Logic and Bit Operations/Compare To Constant  relop===  const=CNobjInvalidID  OutDataTypeStr=boolean
  Logical Operator1 : Logic  Operator=OR  AllPortsSameDT=off  OutDataTypeStr=boolean
  Relational Operator : RelationalOperator  Operator=~=  OutDataTypeStr=boolean  RndMeth=Simplest
  Unit Delay : UnitDelay  SampleTime=-1  HasFrameUpgradeWarning=on
  vehPrefillObjRelAborCondFulfilled : Outport
  wires:
  ObjectID/1 -> Unit Delay/1, Relational Operator/1, Compare To Constant4/1
  Unit Delay/1 -> Relational Operator/2
  Relational Operator/1 -> Logical Operator1/2
  Compare To Constant4/1 -> Logical Operator1/1
  Logical Operator1/1 -> vehPrefillObjRelAborCondFulfilled/1  "vehPrefillObjRelAborCondFulfilled"

== system TosAeb/FcwAebJa_Step_sys/ALG_AEBSystem/ALG_AEBController/AEB_FuncCondCalcApp/LongFunctionCondCalc/vehPrefillSuppAborCondCalc/vehPrefillAborCondCalc/vehPrefillSituationRelAborCondCalc ==
  longCollisionSeverity : Inport
  Compare To Constant1 : simulink/Logic and Bit Operations/Compare To Constant  relop=~=  const=CollisionSeverity.Medium  OutDataTypeStr=boolean
  Compare To Constant2 : simulink/Logic and Bit Operations/Compare To Constant  relop=~=  const=CollisionSeverity.High  OutDataTypeStr=boolean
  Compare To Constant4 : simulink/Logic and Bit Operations/Compare To Constant  relop=~=  const=CollisionSeverity.Low  OutDataTypeStr=boolean
  Logical Operator : Logic  Inputs=3  AllPortsSameDT=off  OutDataTypeStr=boolean
  vehPrefillSituationRelAborCondFulfilled : Outport
  wires:
  Compare To Constant2/1 -> Logical Operator/3
  Compare To Constant4/1 -> Logical Operator/1
  longCollisionSeverity/1 -> Compare To Constant4/1, Compare To Constant2/1, Compare To Constant1/1
  Compare To Constant1/1 -> Logical Operator/2
  Logical Operator/1 -> vehPrefillSituationRelAborCondFulfilled/1

== system TosAeb/FcwAebJa_Step_sys/ALG_AEBSystem/ALG_AEBController/AEB_FuncCondCalcApp/LongFunctionCondCalc/vehPrefillSuppAborCondCalc/vehPrefillAborCondCalc/vehPrefillVehRelAborCondCalc ==
  SteerWheelAngle : Inport
  SteerWheelSpd : Inport  Port=2
  ESPmasterCylindBrakePress : Inport  Port=3
  BrakePedalStatus_G : Inport  Port=4
  AccrPedlPosnDiagc : Inport  Port=5
  vehStateCalcAcuteWarningAborFlg : Inport  Port=6
  DriverAborCondJudge : Subsystem
  vehPreWarningVehRelActiveCondJudge : Subsystem
  vehPrefillVehRelAborCondFulfilled : Outport
  wires:
  AccrPedlPosnDiagc/1 -> DriverAborCondJudge/5(AccrPedlPosnDiagc)
  BrakePedalStatus_G/1 -> DriverAborCondJudge/4(BrakePedalStatus_G)
  ESPmasterCylindBrakePress/1 -> DriverAborCondJudge/3(ESPmasterCylindBrakePress)
  SteerWheelSpd/1 -> DriverAborCondJudge/2(SteerWheelSpd)
  SteerWheelAngle/1 -> DriverAborCondJudge/1(SteerWheelAngle)
  DriverAborCondJudge/1(driverAborCondFlg) -> vehPreWarningVehRelActiveCondJudge/1(driverAborCondFlg)
  vehStateCalcAcuteWarningAborFlg/1 -> vehPreWarningVehRelActiveCondJudge/2(vehStateCalcPrefillAborFlg)
  vehPreWarningVehRelActiveCondJudge/1(vehPrefillVehRelAborCondFulfilled) -> vehPrefillVehRelAborCondFulfilled/1  "vehPrefillVehRelAborCondFulfilled"

== system TosAeb/FcwAebJa_Step_sys/ALG_AEBSystem/ALG_AEBController/AEB_FuncCondCalcApp/LongFunctionCondCalc/vehPrefillSuppAborCondCalc/vehPrefillSuppCondCalc/vehPrefillObjRelActiveCondCalc ==
  ObjectMovingState : Inport
  ObjectTypeCameraConfirmed : Inport  Port=2
  AEB_PedResp : Inport  Port=3
  ObjectType : Inport  Port=4
  subjCurvTraj : Inport  Port=5
  subjLatAccel : Inport  Port=6
  subjVel : Inport  Port=7
  vehConfigAEBCyc : Inport  Port=8
  vehConfigAEBPed : Inport  Port=9
  ObjectMovingOrientation : Inport  Port=10
  ObjectID : Inport  Port=11
  ObjectExistProb : Inport  Port=12
  ObjectIsObstacleProb : Inport  Port=13
  ObjFusQuality : Inport  Port=14
  Compare To Constant : simulink/Logic and Bit Operations/Compare To Constant  relop===  const=AEB_ObjectMovingState.Still  OutDataTypeStr=boolean
  Compare To Constant1 : simulink/Logic and Bit Operations/Compare To Constant  relop===  const=AEB_ObjectMovingState.Moving  OutDataTypeStr=boolean
  Compare To Constant10 : simulink/Logic and Bit Operations/Compare To Constant  relop===  const=TRUE  OutDataTypeStr=boolean
  Compare To Constant12 : simulink/Logic and Bit Operations/Compare To Constant  relop===  const=FALSE  OutDataTypeStr=boolean
  Compare To Constant2 : simulink/Logic and Bit Operations/Compare To Constant  relop===  const=AEB_ObjectMovingState.Stopped  OutDataTypeStr=boolean
  Compare To Constant3 : simulink/Logic and Bit Operations/Compare To Constant  relop===  const=AEB_ObjectMovingOrientation.SameDir  OutDataTypeStr=boolean
  Compare To Constant4 : simulink/Logic and Bit Operations/Compare To Constant  relop=~=  const=CNobjInvalidID  OutDataTypeStr=boolean
  Compare To Constant5 : simulink/Logic and Bit Operations/Compare To Constant  relop=>  const=P_AEB_Func_vehPrefillObjObstacleProbActivationThres  OutDataTypeStr=boolean
  Compare To Constant6 : simulink/Logic and Bit Operations/Compare To Constant  relop=~=  const=CNobjInvalidID  OutDataTypeStr=boolean
  Compare To Constant8 : simulink/Logic and Bit Operations/Compare To Constant  relop===  const=TRUE  OutDataTypeStr=boolean
  Constant1 : Constant  Value=P_AEB_Func_vehPrefillSubjLatAccelActivationThresForPedCyc
  Constant2 : Constant  Value=P_AEB_Func_vehPrefillSubjCurvTrajActivationThresForPedCyc
  Constant3 : Constant  Value=P_AEB_Func_vehPrefillSubjVelActivationThresForPedCyc
  Logical Operator : Logic  Operator=OR  Inputs=3  AllPortsSameDT=off  OutDataTypeStr=boolean
  Logical Operator1 : Logic  AllPortsSameDT=off  OutDataTypeStr=boolean
  Logical Operator2 : Logic  AllPortsSameDT=off  OutDataTypeStr=boolean
  Logical Operator5 : Logic  Inputs=7  AllPortsSameDT=off  OutDataTypeStr=boolean
  PedCycObjCondSuppJudgeFlow : AEBSFunctionLib/PedCycObjCondSuppJudgeFlow  SystemSampleTime=-1  VariantActivationTime=update diagram  Latency=0  AutoFrameSizeCalculation=off
  Product : Product
  Relational Operator : RelationalOperator  Operator===  OutDataTypeStr=boolean  RndMeth=Simplest
  Unit Delay : UnitDelay  SampleTime=-1  HasFrameUpgradeWarning=on
  vehPrefillObjRelActiveCondFulfilled : Outport
  wires:
  Compare To Constant10/1 -> Logical Operator5/7  "ObjFusQuality"
  ObjFusQuality/1 -> Compare To Constant10/1
  Compare To Constant8/1 -> Logical Operator5/2
  Constant1/1 -> PedCycObjCondSuppJudgeFlow/11
  Constant2/1 -> PedCycObjCondSuppJudgeFlow/10
  Constant3/1 -> PedCycObjCondSuppJudgeFlow/9
  AEB_PedResp/1 -> PedCycObjCondSuppJudgeFlow/8
  vehConfigAEBPed/1 -> PedCycObjCondSuppJudgeFlow/7
  vehConfigAEBCyc/1 -> PedCycObjCondSuppJudgeFlow/6
  subjVel/1 -> PedCycObjCondSuppJudgeFlow/5
  subjLatAccel/1 -> PedCycObjCondSuppJudgeFlow/4
  subjCurvTraj/1 -> PedCycObjCondSuppJudgeFlow/3
  ObjectType/1 -> PedCycObjCondSuppJudgeFlow/2
  ObjectTypeCameraConfirmed/1 -> Compare To Constant8/1, PedCycObjCondSuppJudgeFlow/1
  Logical Operator1/1 -> Logical Operator5/5  "objIDCondFulfilled"
  Logical Operator/1 -> Logical Operator5/1  "objMovingStateCondFulfilled"
  PedCycObjCondSuppJudgeFlow/1 -> Compare To Constant12/1  "pedCycObjCondSuppFlg"
  Logical Operator2/1 -> Logical Operator5/6  "objQualityCondFulfilled"
  Compare To Constant3/1 -> Logical Operator5/4  "objMovingOrientationCondFulfilled"
  Logical Operator5/1 -> vehPrefillObjRelActiveCondFulfilled/1  "vehPrefillObjRelActiveCondFulfilled"
  Compare To Constant4/1 -> Logical Operator1/1
  Relational Operator/1 -> Logical Operator1/2
  Compare To Constant/1 -> Logical Operator/3
  Product/1 -> Compare To Constant5/1
  Compare To Constant5/1 -> Logical Operator2/2
  Compare To Constant6/1 -> Logical Operator2/1
  ObjectIsObstacleProb/1 -> Product/2
  ObjectExistProb/1 -> Product/1
  Unit Delay/1 -> Relational Operator/2
  ObjectID/1 -> Compare To Constant4/1, Relational Operator/1, Unit Delay/1, Compare To Constant6/1
  ObjectMovingOrientation/1 -> Compare To Constant3/1
  Compare To Constant1/1 -> Logical Operator/1
  Compare To Constant2/1 -> Logical Operator/2
  ObjectMovingState/1 -> Compare To Constant/1, Compare To Constant2/1, Compare To Constant1/1
  Compare To Constant12/1 -> Logical Operator5/3  "pedCycReleventCondFulfilled"

== system TosAeb/FcwAebJa_Step_sys/ALG_AEBSystem/ALG_AEBController/AEB_FuncCondCalcApp/LongFunctionCondCalc/vehPrefillSuppAborCondCalc/vehPrefillSuppCondCalc/vehPrefillSituationRelActiveCondCalc ==
  longCollisionSeverity : Inport
  overlapRatio : Inport  Port=2
  dxFrontBumper : Inport  Port=3
  probEscape : Inport  Port=4
  Compare To Constant1 : simulink/Logic and Bit Operations/Compare To Constant  relop===  const=CollisionSeverity.Medium  OutDataTypeStr=boolean
  Compare To Constant2 : simulink/Logic and Bit Operations/Compare To Constant  relop===  const=CollisionSeverity.High  OutDataTypeStr=boolean
  Compare To Constant3 : simulink/Logic and Bit Operations/Compare To Constant  relop=>  const=P_AEB_Func_vehPrefillEscapeActivationThres  OutDataTypeStr=boolean
  Compare To Constant4 : simulink/Logic and Bit Operations/Compare To Constant  relop===  const=CollisionSeverity.Low  OutDataTypeStr=boolean
  Compare To Constant5 : simulink/Logic and Bit Operations/Compare To Constant  relop===  const=FALSE  OutDataTypeStr=boolean
  Logical Operator : Logic  Operator=OR  Inputs=3  AllPortsSameDT=off  OutDataTypeStr=boolean
  Logical Operator1 : Logic  Inputs=3  AllPortsSameDT=off  OutDataTypeStr=boolean
  OverlapThesLookupTable : Lookup_n-D  NumberOfTableDimensions=1  BreakpointsForDimension1=P_AEB_Func_vehPrefillOverlapActivationThresX  BeginIndexSearchUsingPreviousIndexResult=on  Table=P_AEB_Func_vehPrefillOverlapActivationThresY  ExtrapMethod=Clip  InternalRulePriority=Speed  RndMeth=Simplest  InputSameDT=on
  Relational Operator : RelationalOperator  Operator=>  OutDataTypeStr=boolean  RndMeth=Simplest
  vehPrefillSituationRelActiveCondFulfilled : Outport
  wires:
  Compare To Constant4/1 -> Logical Operator/1
  Logical Operator1/1 -> vehPrefillSituationRelActiveCondFulfilled/1  "vehPrefillSituationRelActiveCondFulfilled"
  Logical Operator/1 -> Logical Operator1/1  "collisionLvlCondFulfilled"
  Relational Operator/1 -> Logical Operator1/2  "OverlapCondFulfilled"
  Compare To Constant5/1 -> Logical Operator1/3
  Compare To Constant3/1 -> Compare To Constant5/1  "probEscapeFulfilled"
  probEscape/1 -> Compare To Constant3/1
  overlapRatio/1 -> Relational Operator/1
  OverlapThesLookupTable/1 -> Relational Operator/2
  dxFrontBumper/1 -> OverlapThesLookupTable/1
  Compare To Constant2/1 -> Logical Operator/3
  Compare To Constant1/1 -> Logical Operator/2
  longCollisionSeverity/1 -> Compare To Constant4/1, Compare To Constant2/1, Compare To Constant1/1

== system TosAeb/FcwAebJa_Step_sys/ALG_AEBSystem/ALG_AEBController/AEB_FuncCondCalcApp/LongFunctionCondCalc/vehPrefillSuppAborCondCalc/vehPrefillSuppCondCalc/vehPrefillSuppCondFinalJudge ==
  vehPrefillObjRelActiveCondFulfilled : Inport
  vehPrefillVehRelActiveCondFulfilled : Inport  Port=2
  vehPrefillSituationRelActiveCondFulfilled : Inport  Port=3
  Compare To Constant1 : simulink/Logic and Bit Operations/Compare To Constant  relop===  const=FALSE  OutDataTypeStr=boolean
  Compare To Constant2 : simulink/Logic and Bit Operations/Compare To Constant  relop===  const=FALSE  OutDataTypeStr=boolean
  Compare To Constant5 : simulink/Logic and Bit Operations/Compare To Constant  relop===  const=FALSE  OutDataTypeStr=boolean
  Logical Operator : Logic  Operator=OR  Inputs=3  AllPortsSameDT=off  OutDataTypeStr=boolean
  vehPrefillSuppCondFulfilled : Outport
  wires:
  Compare To Constant2/1 -> Logical Operator/3
  Compare To Constant1/1 -> Logical Operator/2
  Compare To Constant5/1 -> Logical Operator/1
  Logical Operator/1 -> vehPrefillSuppCondFulfilled/1  "vehPrefillSuppCondFulfilled"
  vehPrefillSituationRelActiveCondFulfilled/1 -> Compare To Constant2/1
  vehPrefillObjRelActiveCondFulfilled/1 -> Compare To Constant5/1
  vehPrefillVehRelActiveCondFulfilled/1 -> Compare To Constant1/1

== system TosAeb/FcwAebJa_Step_sys/ALG_AEBSystem/ALG_AEBController/AEB_FuncCondCalcApp/LongFunctionCondCalc/vehPrefillSuppAborCondCalc/vehPrefillSuppCondCalc/vehPrefillVehRelActiveCondCalc ==
  subjVel : Inport
  ObjectMovingState : Inport  Port=2
  SteerWheelAngle : Inport  Port=3
  SteerWheelSpd : Inport  Port=4
  VehConfigIbooster : Inport  Port=5
  ESPmasterCylindBrakePress : Inport  Port=6
  BrakePedalStatus_G : Inport  Port=7
  IB_BrkPedalAppld : Inport  Port=8
  AccrPedlPosnDiagc : Inport  Port=9
  vehStateCalcPrefillSuppFlg : Inport  Port=10
  DriverCondJudge : Subsystem
  subjVelWork : Subsystem
  vehAcuteWarningVehRelActiveCondJudge : Subsystem
  vehPrefillVehRelActiveCondFulfilled : Outport
  wires:
  VehConfigIbooster/1 -> DriverCondJudge/3(VehConfigIbooster)
  IB_BrkPedalAppld/1 -> DriverCondJudge/6(IB_BrkPedalAppld)
  AccrPedlPosnDiagc/1 -> DriverCondJudge/7(AccrPedlPosnDiagc)
  BrakePedalStatus_G/1 -> DriverCondJudge/5(BrakePedalStatus_G)
  ESPmasterCylindBrakePress/1 -> DriverCondJudge/4(ESPmasterCylindBrakePress)
  SteerWheelSpd/1 -> DriverCondJudge/2(SteerWheelSpd)
  SteerWheelAngle/1 -> DriverCondJudge/1(SteerWheelAngle)
  vehAcuteWarningVehRelActiveCondJudge/1(vehPrefillVehRelActiveCondFulfilled) -> vehPrefillVehRelActiveCondFulfilled/1  "vehPrefillVehRelActiveCondFulfilled"
  vehStateCalcPrefillSuppFlg/1 -> vehAcuteWarningVehRelActiveCondJudge/5(vehStateCalcPrefillSuppFlg)
  subjVel/1 -> vehAcuteWarningVehRelActiveCondJudge/1(subjVel)
  DriverCondJudge/1(driverSuppCondFlg) -> vehAcuteWarningVehRelActiveCondJudge/4(driverSuppCondFlg)
  subjVelWork/2(subjVelWorkMin) -> vehAcuteWarningVehRelActiveCondJudge/3(subjVelWorkMin)
  subjVelWork/1(subjVelWorkMax) -> vehAcuteWarningVehRelActiveCondJudge/2(subjVelWorkMax)
  ObjectMovingState/1 -> subjVelWork/1(ObjectMovingState)

== system TosAeb/FcwAebJa_Step_sys/ALG_AEBSystem/ALG_AEBController/AEB_FuncCondCalcApp/PedFunctionCondCalc/pedABASuppAborCond/pedABAAborCondCalc/pedABAAborCondFinalJudge ==
  pedABAObjRelAborCondFulfilled : Inport
  pedABAVehRelAborCondFulfilled : Inport  Port=2
  pedABASituationRelAborCondFulfilled : Inport  Port=3
  Compare To Constant1 : simulink/Logic and Bit Operations/Compare To Constant  relop===  const=TRUE  OutDataTypeStr=boolean
  Compare To Constant2 : simulink/Logic and Bit Operations/Compare To Constant  relop===  const=TRUE  OutDataTypeStr=boolean
  Compare To Constant3 : simulink/Logic and Bit Operations/Compare To Constant  relop===  const=TRUE  OutDataTypeStr=boolean
  Logical Operator : Logic  Operator=OR  Inputs=3  AllPortsSameDT=off  OutDataTypeStr=boolean
  pedABAAborCondFulfilled : Outport
  wires:
  Compare To Constant2/1 -> Logical Operator/3
  Compare To Constant1/1 -> Logical Operator/2
  pedABAObjRelAborCondFulfilled/1 -> Compare To Constant3/1
  Logical Operator/1 -> pedABAAborCondFulfilled/1  "vehPreWarningAborCondFulfilled"
  pedABASituationRelAborCondFulfilled/1 -> Compare To Constant2/1
  pedABAVehRelAborCondFulfilled/1 -> Compare To Constant1/1
  Compare To Constant3/1 -> Logical Operator/1

== system TosAeb/FcwAebJa_Step_sys/ALG_AEBSystem/ALG_AEBController/AEB_FuncCondCalcApp/PedFunctionCondCalc/pedABASuppAborCond/pedABAAborCondCalc/pedABAObjRelActiveCondCalc ==
  ObjectID : Inport
  Compare To Constant4 : simulink/Logic and Bit Operations/Compare To Constant  relop===  const=CNobjInvalidID  OutDataTypeStr=boolean
  pedABAObjRelAborCondFulfilled : Outport
  wires:
  ObjectID/1 -> Compare To Constant4/1
  Compare To Constant4/1 -> pedABAObjRelAborCondFulfilled/1

== system TosAeb/FcwAebJa_Step_sys/ALG_AEBSystem/ALG_AEBController/AEB_FuncCondCalcApp/PedFunctionCondCalc/pedABASuppAborCond/pedABAAborCondCalc/pedABASituationRelAborCondCalc ==
  note: 将true改为false
  latCollisionSeverity : Inport
  pedABAWorkTime : Inport  Port=2
  Compare To Constant : simulink/Logic and Bit Operations/Compare To Constant  relop===  const=CollisionSeverity.None  OutDataTypeStr=boolean
  Compare To Constant1 : simulink/Logic and Bit Operations/Compare To Constant  relop=>=  const=P_AEB_Func_pedABAMaxActivationTimeAborThres  OutDataTypeStr=boolean
  Logical Operator : Logic  AllPortsSameDT=off  OutDataTypeStr=boolean
  pedABASituationRelAborCondFulfilled : Outport
  wires:
  Logical Operator/1 -> pedABASituationRelAborCondFulfilled/1
  latCollisionSeverity/1 -> Compare To Constant/1
  Compare To Constant/1 -> Logical Operator/1
  pedABAWorkTime/1 -> Compare To Constant1/1
  Compare To Constant1/1 -> Logical Operator/2

== system TosAeb/FcwAebJa_Step_sys/ALG_AEBSystem/ALG_AEBController/AEB_FuncCondCalcApp/PedFunctionCondCalc/pedABASuppAborCond/pedABAAborCondCalc/pedABAVehRelAborCondCalc ==
  note: 取消速度限制
  vehStateCalcABAAborFlg : Inport
  latCollisionSeverity : Inport  Port=2
  SteerWheelAngle : Inport  Port=3
  SteerWheelSpd : Inport  Port=4
  Abs : Abs
  Abs1 : Abs
  Compare To Constant : simulink/Logic and Bit Operations/Compare To Constant  relop===  const=CollisionSeverity.None  OutDataTypeStr=boolean
  Compare To Constant1 : simulink/Logic and Bit Operations/Compare To Constant  relop=>  const=P_AEB_Func_pedABAStrWheelAngleAborThres  OutDataTypeStr=boolean
  Compare To Constant2 : simulink/Logic and Bit Operations/Compare To Constant  relop=>  const=P_AEB_Func_pedABAStrWheelAngleSpdAborThres  OutDataTypeStr=boolean
  Compare To Constant3 : simulink/Logic and Bit Operations/Compare To Constant  relop===  const=TRUE  OutDataTypeStr=boolean
  Logical Operator2 : Logic  Operator=OR  AllPortsSameDT=off  OutDataTypeStr=boolean
  Logical Operator3 : Logic  AllPortsSameDT=off  OutDataTypeStr=boolean
  Logical Operator4 : Logic  Operator=OR  AllPortsSameDT=off  OutDataTypeStr=boolean
  pedABAVehRelAborCondFulfilled : Outport
  wires:
  Logical Operator3/1 -> Logical Operator2/2  "strReleventSuppCondFulfilled"
  Logical Operator4/1 -> Logical Operator3/2
  SteerWheelSpd/1 -> Abs1/1
  Abs/1 -> Compare To Constant1/1
  Compare To Constant2/1 -> Logical Operator4/2
  Compare To Constant1/1 -> Logical Operator4/1
  SteerWheelAngle/1 -> Abs/1
  Abs1/1 -> Compare To Constant2/1
  Compare To Constant/1 -> Logical Operator3/1
  latCollisionSeverity/1 -> Compare To Constant/1
  Compare To Constant3/1 -> Logical Operator2/1  "vehStateCalcPrefillAborCondFulfilled"
  vehStateCalcABAAborFlg/1 -> Compare To Constant3/1
  Logical Operator2/1 -> pedABAVehRelAborCondFulfilled/1
